simulink model "Lab2_simulink_plc"  (R2023b)
settings: Solver=FixedStepAuto  StartTime=0.0  StopTime=inf  RelTol=1e-3
InitFcn:
  | d13_init.PRE = 50;
undefined symbols (not defined in the file or in its folder): BOOL, DINT, OTL, slplc
libraries: arduinolib x9
(part 6 of 31)

== system Lab2_simulink_plc/PLC Controller Suite/__Logic/IR sampling/IR sampling/__Logic ==
  note: Continuosly sample the IR sensor
  Add Rungs : Subsystem  DisableCoverage=on  TreatAsAtomicUnit=on  mask{PLCRungNumberToAdd=2, PLCBlockType=AddRungs}
      mask prompts: PLCRungNumberToAdd "Rung Number:"
      mask Help: web([docroot '/plccoder/ref/addrungs' '.html']);
      mask DialogControl PLCAddRungs Prompt: Add Rungs
      mask DialogControl PLCRungAddButton Prompt: Add
      mask DialogControl PLCRungAddButton Callback: slplc.callbacks.addLadderRungs(gcb);
      mask Display: disp(sprintf(['Add' newline 'Rungs']));
  Add Single Rung : Subsystem  DisableCoverage=on  TreatAsAtomicUnit=on  mask{PLCRungNumberToAdd=1, PLCBlockType=AddSingleRung}
      settings: OpenFcn=slplc.callbacks.addLadderRungs(gcb);
      mask Help: web([docroot '/plccoder/ref/addsinglerung' '.html']);
      mask DialogControl PLCAddRung Prompt: Add Single Rung
      mask Display: disp(sprintf(['Add' newline '1 Rung']));
  MOV : Subsystem  Priority=3  TreatAsAtomicUnit=on  mask{PLCBlockType=MOV, PLCPOUType=stdFC}
      mask Help: web([docroot '/plccoder/ref/mov' '.html']);
      mask DialogControl PLCBlockTitle Prompt: Move (MOV)
      mask DialogControl PLCBlockDesc Prompt: Move a copy of the Source to the Destination. The Source remains unchanged.
      mask Display: disp('MOV');
  Power Rail Start : Subsystem  Priority=1  DisableCoverage=on  TreatAsAtomicUnit=on  mask{PLCBlockType=PowerRailStart}
      mask Help: web([docroot '/plccoder/ref/powerrailstart' '.html']);
      mask DialogControl PLCBlockTitle Prompt: Power Rail Start
      mask DialogControl PLCBlockDesc Prompt: This is the ladder diagram left power rail start block.
  Power Rail Terminal1 : Subsystem  DisableCoverage=on  TreatAsAtomicUnit=on  mask{PLCBlockType=PowerRailTerminal}
      mask Help: web([docroot '/plccoder/ref/powerrailterminal' '.html']);
      mask DialogControl PLCBlockTitle Prompt: Power Rail Terminal
      mask DialogControl PLCBlockDesc Prompt: This is the ladder diagram left power rail terminal block.
  Program Variables : Subsystem  Tag=ProgramVariableSS  DisableCoverage=on  TreatAsAtomicUnit=on  mask{PLCVarIR_valueName=IR_value, PLCVarIR_valueScope=External, PLCVarIR_valuePortType=Hidden, PLCVarIR_valuePortIndex=2, PLCVarIR_valueDataType=DINT, PLCVarIR_valueSize=-1, PLCVarIR_valueInitialValue=0, PLCVarIR_valueToDelete=off, PLCVarIR_value_copyName=IR_value_copy, PLCVarIR_value_copyScope=External, PLCVarIR_value_copyPortType=Hidden, PLCVarIR_value_copyPortIndex=2, PLCVarIR_value_copyDataType=DINT, PLCVarIR_value_copySize=-1, PLCVarIR_value_copyInitialValue=0, PLCVarIR_value_copyToDelete=off}
      mask init:
      | slplc.callbacks.initPOUVarSS(gcb);
      settings: OpenFcn=slplc.callbacks.openProgramVariableSS(gcb);
      mask Help: web([docroot '/plccoder/ref/programvariables' '.html']);
      mask TypeOptions PLCVarIR_valueScope: Local | External
      mask Callback PLCVarIR_valueScope: slplc.callbacks.updatePOUVarSS(gcb);
      mask TypeOptions PLCVarIR_valuePortType: Hidden | Inport | Outport
      mask Callback PLCVarIR_valuePortType: slplc.callbacks.updatePOUVarSS(gcb);
      mask TypeOptions PLCVarIR_valuePortIndex: 1 | 2
      mask TypeOptions PLCVarIR_value_copyScope: Local | External
      mask Callback PLCVarIR_value_copyScope: slplc.callbacks.updatePOUVarSS(gcb);
      mask TypeOptions PLCVarIR_value_copyPortType: Hidden | Inport | Outport
      mask Callback PLCVarIR_value_copyPortType: slplc.callbacks.updatePOUVarSS(gcb);
      mask TypeOptions PLCVarIR_value_copyPortIndex: 1 | 2
      mask DialogControl PLCBlockTitle Prompt: Program Variable Spreadsheet
      mask DialogControl PLCVarListHeaderName Prompt: Name
      mask DialogControl PLCVarListHeaderScope Prompt: Scope
      mask DialogControl PLCVarListHeaderDataType Prompt: Data Type
      mask DialogControl PLCVarListHeaderSize Prompt: Size
      mask DialogControl PLCVarListHeaderInitialValue Prompt: Initial Value
      mask DialogControl PLCVarListHeaderToDelete Prompt: Delete
  Rung Terminal1 : Subsystem  Priority=5  DisableCoverage=on  TreatAsAtomicUnit=on  mask{PLCRungTerminalIndex=1, PLCBlockType=RungTerminal}
      settings: AttributesFormatString=Rung %<PLCRungTerminalIndex>
      mask Help: web([docroot '/plccoder/ref/rungterminal' '.html']);
      mask DialogControl PLCBlockTitle Prompt: Rung Terminal
      mask DialogControl PLCBlockDescText Prompt: This is the ladder diagram rung terminal.
  Up to Parent POU : Subsystem  DisableCoverage=on  TreatAsAtomicUnit=on  mask{PLCBlockType=OpenParentPOU}
      settings: OpenFcn=slplc.callbacks.openParentPOUBlock(gcb);
      mask Help: web([docroot '/plccoder/ref/uptoparentpou' '.html']);
      mask DialogControl PLCBlockTitle Prompt: Add Single Rung
  Variable Read : Subsystem  Priority=2  TreatAsAtomicUnit=on  mask{PLCOperandTag=IR_value, PLCBlockType=VariableRead, PLCOperandAccess=Read, PLCPOUType=VariableRead, PLCOperandExpression=xxx_PLC_VAR_IR_value}
      mask prompts: PLCOperandTag "Data Tag:"
      mask init:
      | block = gcb;
      | maskObj = Simulink.Mask.get(block);
      | maskObj.Display = sprintf('disp(''%s'');', PLCOperandTag);
      | slplc.callbacks.initInstruction(block);
      mask Help: web([docroot '/plccoder/ref/variableread' '.html']);
      mask DialogControl PLCBlockTitle Prompt: Data Tag Read
      mask Display: disp('IR_value');
  Variable Write : Subsystem  Priority=4  TreatAsAtomicUnit=on  mask{PLCOperandTag=IR_value_copy, PLCBlockType=VariableWrite, PLCOperandAccess=Write, PLCPOUType=VariableWrite, PLCOperandExpression=xxx_PLC_VAR_IR_value_copy}
      mask prompts: PLCOperandTag "Data Tag:"
      mask init: same as Lab2_simulink_plc/PLC Controller Suite/__Logic/Fukt logic/Program/__Logic/Variable Write
      mask Help: web([docroot '/plccoder/ref/variablewrite' '.html']);
      mask DialogControl PLCBlockTitle Prompt: Data Tag Write
      mask Display: disp('IR_value_copy');
  wires:
  Power Rail Start/1(PowerOut) -> Power Rail Terminal1/1(PowerIn), MOV/1(RungIn)
  MOV/1(RungOut) -> Rung Terminal1/1(RungIn)
  Variable Read/1(VarOut) -> MOV/2(src)
  MOV/2(dest) -> Variable Write/1(VarIn)

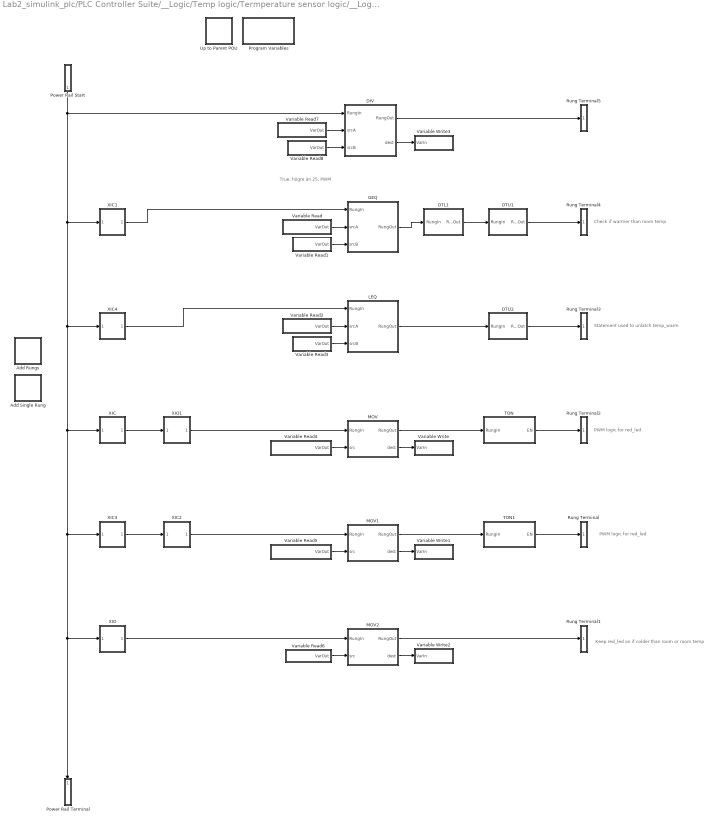
== system Lab2_simulink_plc/PLC Controller Suite/__Logic/Temp logic/Termperature sensor logic/__Logic ==
  note: Keep red_led on if colder than room or room temp
  note: PWM logic for red_led
  note: Check if warmer than room temp
  note: Statement used to unlatch temp_warm
  note: True, högre än 25, PWM
  note: PWM logic for red_led
  Add Rungs : Subsystem  DisableCoverage=on  TreatAsAtomicUnit=on  mask{PLCRungNumberToAdd=2, PLCBlockType=AddRungs}
      mask prompts: PLCRungNumberToAdd "Rung Number:"
      mask Help: web([docroot '/plccoder/ref/addrungs' '.html']);
      mask DialogControl PLCAddRungs Prompt: Add Rungs
      mask DialogControl PLCRungAddButton Prompt: Add
      mask DialogControl PLCRungAddButton Callback: slplc.callbacks.addLadderRungs(gcb);
      mask Display: disp(sprintf(['Add' newline 'Rungs']));
  Add Single Rung : Subsystem  DisableCoverage=on  TreatAsAtomicUnit=on  mask{PLCRungNumberToAdd=1, PLCBlockType=AddSingleRung}
      settings: OpenFcn=slplc.callbacks.addLadderRungs(gcb);
      mask Help: web([docroot '/plccoder/ref/addsinglerung' '.html']);
      mask DialogControl PLCAddRung Prompt: Add Single Rung
      mask Display: disp(sprintf(['Add' newline '1 Rung']));
  DIV : Subsystem  Priority=4  TreatAsAtomicUnit=on  mask{PLCBlockType=DIV, PLCPOUType=stdFC}
      mask Help: web([docroot '/plccoder/ref/div' '.html']);
      mask DialogControl PLCBlockTitle Prompt: Divide (DIV)
      mask DialogControl PLCBlockDesc Prompt: Divide Source A by Source B.
      mask Display: disp('DIV');
  GEQ : Subsystem  Priority=10  TreatAsAtomicUnit=on  mask{PLCBlockType=GEQ, PLCPOUType=stdFC}
      mask Help: web([docroot '/plccoder/ref/geq' '.html']);
      mask DialogControl PLCBlockTitle Prompt: Greater Than or Equal To (GEQ)
      mask DialogControl PLCBlockDesc Prompt: Test whether Source A is greater than or equal to Source B.
      mask Display: disp('GEQ');
  LEQ : Subsystem  Priority=17  TreatAsAtomicUnit=on  mask{PLCBlockType=LEQ, PLCPOUType=stdFC}
      mask Help: web([docroot '/plccoder/ref/leq' '.html']);
      mask DialogControl PLCBlockTitle Prompt: Less Than or Equal To (LEQ)
      mask DialogControl PLCBlockDesc Prompt: Test whether Source A is less than or equal to Source B.
      mask Display: disp('LEQ');
  MOV : Subsystem  Priority=23  TreatAsAtomicUnit=on  mask{PLCBlockType=MOV, PLCPOUType=stdFC}
      mask Help: web([docroot '/plccoder/ref/mov' '.html']);
      mask DialogControl PLCBlockTitle Prompt: Move (MOV)
      mask DialogControl PLCBlockDesc Prompt: Move a copy of the Source to the Destination. The Source remains unchanged.
      mask Display: disp('MOV');
  MOV1 : Subsystem  Priority=30  TreatAsAtomicUnit=on  mask{PLCBlockType=MOV, PLCPOUType=stdFC}
      mask Help: web([docroot '/plccoder/ref/mov' '.html']);
      mask DialogControl PLCBlockTitle Prompt: Move (MOV)
      mask DialogControl PLCBlockDesc Prompt: Move a copy of the Source to the Destination. The Source remains unchanged.
      mask Display: disp('MOV');
  MOV2 : Subsystem  Priority=36  TreatAsAtomicUnit=on  mask{PLCBlockType=MOV, PLCPOUType=stdFC}
      mask Help: web([docroot '/plccoder/ref/mov' '.html']);
      mask DialogControl PLCBlockTitle Prompt: Move (MOV)
      mask DialogControl PLCBlockDesc Prompt: Move a copy of the Source to the Destination. The Source remains unchanged.
      mask Display: disp('MOV');
  OTL1 : Subsystem  Priority=11  TreatAsAtomicUnit=on  mask{PLCOperandTag=temp_warm, PLCBlockType=OTL, PLCPOUType=Coil, PLCOperandExpression=xxx_PLC_VAR_temp_warm}
      mask prompts: PLCOperandTag "Operand Tag:"
      mask init:
      | slplc.callbacks.initInstruction(gcb);
      settings: AttributesFormatString=%<PLCOperandTag>
      mask Help: web([docroot '/plccoder/ref/otl' '.html']);
      mask DialogControl PLCBlockTitle Prompt: Output Latch (OTL)
      mask Display: disp('L');
  OTU1 : Subsystem  Priority=12  TreatAsAtomicUnit=on  mask{PLCOperandTag=TempPolling, PLCBlockType=OTU, PLCPOUType=Coil, PLCOperandExpression=xxx_PLC_VAR_TempPolling}
      mask prompts: PLCOperandTag "Operand Tag:"
      mask init:
      | slplc.callbacks.initInstruction(gcb);
      settings: AttributesFormatString=%<PLCOperandTag>
      mask Help: web([docroot '/plccoder/ref/otu' '.html']);
      mask DialogControl PLCBlockTitle Prompt: Output Unlatch (OTU)
      mask Display: disp('U');
  OTU2 : Subsystem  Priority=18  TreatAsAtomicUnit=on  mask{PLCOperandTag=temp_warm, PLCBlockType=OTU, PLCPOUType=Coil, PLCOperandExpression=xxx_PLC_VAR_temp_warm}
      mask prompts: PLCOperandTag "Operand Tag:"
      mask init:
      | slplc.callbacks.initInstruction(gcb);
      settings: AttributesFormatString=%<PLCOperandTag>
      mask Help: web([docroot '/plccoder/ref/otu' '.html']);
      mask DialogControl PLCBlockTitle Prompt: Output Unlatch (OTU)
      mask Display: disp('U');
  Power Rail Start : Subsystem  Priority=1  DisableCoverage=on  TreatAsAtomicUnit=on  mask{PLCBlockType=PowerRailStart}
      mask Help: web([docroot '/plccoder/ref/powerrailstart' '.html']);
      mask DialogControl PLCBlockTitle Prompt: Power Rail Start
      mask DialogControl PLCBlockDesc Prompt: This is the ladder diagram left power rail start block.
  Power Rail Terminal : Subsystem  Priority=14  DisableCoverage=on  TreatAsAtomicUnit=on  mask{PLCBlockType=PowerRailTerminal}
      mask Help: web([docroot '/plccoder/ref/powerrailterminal' '.html']);
      mask DialogControl PLCBlockTitle Prompt: Power Rail Terminal
      mask DialogControl PLCBlockDesc Prompt: This is the ladder diagram left power rail terminal block.
  Program Variables : Subsystem  Tag=ProgramVariableSS  DisableCoverage=on  TreatAsAtomicUnit=on  mask{PLCVarRoom_tempName=Room_temp, PLCVarRoom_tempScope=Local, PLCVarRoom_tempPortType=Hidden, PLCVarRoom_tempPortIndex=10, PLCVarRoom_tempDataType=uint16, PLCVarRoom_tempSize=1, PLCVarRoom_tempInitialValue=150, PLCVarRoom_tempToDelete=off, PLCVarT1Name=T1, PLCVarT1Scope=Local, PLCVarT1PortType=Hidden, PLCVarT1PortIndex=10, PLCVarT1DataType=Bus: TIMER, PLCVarT1Size=1, PLCVarT1InitialValue=red_period, PLCVarT1ToDelete=off, PLCVarT2Name=T2, PLCVarT2Scope=Local, PLCVarT2PortType=Hidden, PLCVarT2PortIndex=10, PLCVarT2DataType=Bus: TIMER, PLCVarT2Size=1, PLCVarT2InitialValue=red_period, PLCVarT2ToDelete=off, PLCVarconstantName=constant, PLCVarconstantScope=Local, PLCVarconstantPortType=Hidden, PLCVarconstantPortIndex=10, PLCVarconstantDataType=uint16, PLCVarconstantSize=1, PLCVarconstantInitialValue=3, PLCVarconstantToDelete=off, PLCVarduty_cycleName=duty_cycle, PLCVarduty_cycleScope=Local, PLCVarduty_cyclePortType=Hidden, PLCVarduty_cyclePortIndex=10, PLCVarduty_cycleDataType=uint8, PLCVarduty_cycleSize=1, PLCVarduty_cycleInitialValue=0, PLCVarduty_cycleToDelete=off, PLCVarred_led_offName=red_led_off, PLCVarred_led_offScope=Local, PLCVarred_led_offPortType=Hidden, PLCVarred_led_offPortIndex=10, PLCVarred_led_offDataType=uint8, PLCVarred_led_offSize=1, PLCVarred_led_offInitialValue=0, PLCVarred_led_offToDelete=off, PLCVarRedLedName=RedLed, PLCVarRedLedScope=External, PLCVarRedLedPortType=Hidden, PLCVarRedLedPortIndex=10, PLCVarRedLedDataType=DINT, PLCVarRedLedSize=-1, PLCVarRedLedInitialValue=0, PLCVarRedLedToDelete=off, PLCVarTempPollingName=TempPolling, PLCVarTempPollingScope=External, PLCVarTempPollingPortType=Hidden, PLCVarTempPollingPortIndex=10, PLCVarTempPollingDataType=DINT, PLCVarTempPollingSize=-1, PLCVarTempPollingInitialValue=0, PLCVarTempPollingToDelete=off, PLCVartemp_sensor_copyName=temp_sensor_copy, PLCVartemp_sensor_copyScope=External, PLCVartemp_sensor_copyPortType=Hidden, PLCVartemp_sensor_copyPortIndex=10, PLCVartemp_sensor_copyDataType=DINT, PLCVartemp_sensor_copySize=-1, PLCVartemp_sensor_copyInitialValue=0, PLCVartemp_sensor_copyToDelete=off, PLCVartemp_warmName=temp_warm, PLCVartemp_warmScope=External, PLCVartemp_warmPortType=Hidden, PLCVartemp_warmPortIndex=10, PLCVartemp_warmDataType=DINT, PLCVartemp_warmSize=-1, PLCVartemp_warmInitialValue=0, PLCVartemp_warmToDelete=off}
      mask init:
      | slplc.callbacks.initPOUVarSS(gcb);
      settings: OpenFcn=slplc.callbacks.openProgramVariableSS(gcb);
      mask Help: web([docroot '/plccoder/ref/programvariables' '.html']);
      mask TypeOptions PLCVarRoom_tempScope: Local | External
      mask Callback PLCVarRoom_tempScope: slplc.callbacks.updatePOUVarSS(gcb);
      mask TypeOptions PLCVarRoom_tempPortType: Hidden | Inport | Outport
      mask Callback PLCVarRoom_tempPortType: slplc.callbacks.updatePOUVarSS(gcb);
      mask TypeOptions PLCVarRoom_tempPortIndex: 1 | 2 | 3 | 4 | 5 | 6 | 7 | 8 | 9 | 10
      mask TypeOptions PLCVarT1Scope: Local | External
      mask Callback PLCVarT1Scope: slplc.callbacks.updatePOUVarSS(gcb);
      mask TypeOptions PLCVarT1PortType: Hidden | Inport | Outport
      mask Callback PLCVarT1PortType: slplc.callbacks.updatePOUVarSS(gcb);
      mask TypeOptions PLCVarT1PortIndex: 1 | 2 | 3 | 4 | 5 | 6 | 7 | 8 | 9 | 10
      mask TypeOptions PLCVarT2Scope: Local | External
      mask Callback PLCVarT2Scope: slplc.callbacks.updatePOUVarSS(gcb);
      mask TypeOptions PLCVarT2PortType: Hidden | Inport | Outport
      mask Callback PLCVarT2PortType: slplc.callbacks.updatePOUVarSS(gcb);
      mask TypeOptions PLCVarT2PortIndex: 1 | 2 | 3 | 4 | 5 | 6 | 7 | 8 | 9 | 10
      mask TypeOptions PLCVarconstantScope: Local | External
      mask Callback PLCVarconstantScope: slplc.callbacks.updatePOUVarSS(gcb);
      mask TypeOptions PLCVarconstantPortType: Hidden | Inport | Outport
      mask Callback PLCVarconstantPortType: slplc.callbacks.updatePOUVarSS(gcb);
      mask TypeOptions PLCVarconstantPortIndex: 1 | 2 | 3 | 4 | 5 | 6 | 7 | 8 | 9 | 10
      mask TypeOptions PLCVarduty_cycleScope: Local | External
      mask Callback PLCVarduty_cycleScope: slplc.callbacks.updatePOUVarSS(gcb);
      mask TypeOptions PLCVarduty_cyclePortType: Hidden | Inport | Outport
      mask Callback PLCVarduty_cyclePortType: slplc.callbacks.updatePOUVarSS(gcb);
      mask TypeOptions PLCVarduty_cyclePortIndex: 1 | 2 | 3 | 4 | 5 | 6 | 7 | 8 | 9 | 10
      mask TypeOptions PLCVarred_led_offScope: Local | External
      mask Callback PLCVarred_led_offScope: slplc.callbacks.updatePOUVarSS(gcb);
      mask TypeOptions PLCVarred_led_offPortType: Hidden | Inport | Outport
      mask Callback PLCVarred_led_offPortType: slplc.callbacks.updatePOUVarSS(gcb);
      mask TypeOptions PLCVarred_led_offPortIndex: 1 | 2 | 3 | 4 | 5 | 6 | 7 | 8 | 9 | 10
      mask TypeOptions PLCVarRedLedScope: Local | External
      mask Callback PLCVarRedLedScope: slplc.callbacks.updatePOUVarSS(gcb);
      mask TypeOptions PLCVarRedLedPortType: Hidden | Inport | Outport
      mask Callback PLCVarRedLedPortType: slplc.callbacks.updatePOUVarSS(gcb);
      mask TypeOptions PLCVarRedLedPortIndex: 1 | 2 | 3 | 4 | 5 | 6 | 7 | 8 | 9 | 10
      mask TypeOptions PLCVarTempPollingScope: Local | External
      mask Callback PLCVarTempPollingScope: slplc.callbacks.updatePOUVarSS(gcb);
      mask TypeOptions PLCVarTempPollingPortType: Hidden | Inport | Outport
      mask Callback PLCVarTempPollingPortType: slplc.callbacks.updatePOUVarSS(gcb);
      mask TypeOptions PLCVarTempPollingPortIndex: 1 | 2 | 3 | 4 | 5 | 6 | 7 | 8 | 9 | 10
      mask TypeOptions PLCVartemp_sensor_copyScope: Local | External
      mask Callback PLCVartemp_sensor_copyScope: slplc.callbacks.updatePOUVarSS(gcb);
      mask TypeOptions PLCVartemp_sensor_copyPortType: Hidden | Inport | Outport
      mask Callback PLCVartemp_sensor_copyPortType: slplc.callbacks.updatePOUVarSS(gcb);
      mask TypeOptions PLCVartemp_sensor_copyPortIndex: 1 | 2 | 3 | 4 | 5 | 6 | 7 | 8 | 9 | 10
      mask TypeOptions PLCVartemp_warmScope: Local | External
      mask Callback PLCVartemp_warmScope: slplc.callbacks.updatePOUVarSS(gcb);
      mask TypeOptions PLCVartemp_warmPortType: Hidden | Inport | Outport
      mask Callback PLCVartemp_warmPortType: slplc.callbacks.updatePOUVarSS(gcb);
      mask TypeOptions PLCVartemp_warmPortIndex: 1 | 2 | 3 | 4 | 5 | 6 | 7 | 8 | 9 | 10
      mask DialogControl PLCBlockTitle Prompt: Program Variable Spreadsheet
      mask DialogControl PLCVarListHeaderName Prompt: Name
      mask DialogControl PLCVarListHeaderScope Prompt: Scope
      mask DialogControl PLCVarListHeaderDataType Prompt: Data Type
      mask DialogControl PLCVarListHeaderSize Prompt: Size
      mask DialogControl PLCVarListHeaderInitialValue Prompt: Initial Value
      mask DialogControl PLCVarListHeaderToDelete Prompt: Delete
  Rung Terminal : Subsystem  Priority=33  DisableCoverage=on  TreatAsAtomicUnit=on  mask{PLCRungTerminalIndex=5, PLCBlockType=RungTerminal}
      settings: AttributesFormatString=Rung %<PLCRungTerminalIndex>
      mask Help: web([docroot '/plccoder/ref/rungterminal' '.html']);
      mask DialogControl PLCBlockTitle Prompt: Rung Terminal
      mask DialogControl PLCBlockDescText Prompt: This is the ladder diagram rung terminal.
  Rung Terminal1 : Subsystem  Priority=38  DisableCoverage=on  TreatAsAtomicUnit=on  mask{PLCRungTerminalIndex=6, PLCBlockType=RungTerminal}
      settings: AttributesFormatString=Rung %<PLCRungTerminalIndex>
      mask Help: web([docroot '/plccoder/ref/rungterminal' '.html']);
      mask DialogControl PLCBlockTitle Prompt: Rung Terminal
      mask DialogControl PLCBlockDescText Prompt: This is the ladder diagram rung terminal.
  Rung Terminal2 : Subsystem  Priority=26  DisableCoverage=on  TreatAsAtomicUnit=on  mask{PLCRungTerminalIndex=4, PLCBlockType=RungTerminal}
      settings: AttributesFormatString=Rung %<PLCRungTerminalIndex>
      mask Help: web([docroot '/plccoder/ref/rungterminal' '.html']);
      mask DialogControl PLCBlockTitle Prompt: Rung Terminal
      mask DialogControl PLCBlockDescText Prompt: This is the ladder diagram rung terminal.
  Rung Terminal3 : Subsystem  Priority=19  DisableCoverage=on  TreatAsAtomicUnit=on  mask{PLCRungTerminalIndex=3, PLCBlockType=RungTerminal}
      settings: AttributesFormatString=Rung %<PLCRungTerminalIndex>
      mask Help: web([docroot '/plccoder/ref/rungterminal' '.html']);
      mask DialogControl PLCBlockTitle Prompt: Rung Terminal
      mask DialogControl PLCBlockDescText Prompt: This is the ladder diagram rung terminal.
  Rung Terminal4 : Subsystem  Priority=13  DisableCoverage=on  TreatAsAtomicUnit=on  mask{PLCRungTerminalIndex=2, PLCBlockType=RungTerminal}
      settings: AttributesFormatString=Rung %<PLCRungTerminalIndex>
      mask Help: web([docroot '/plccoder/ref/rungterminal' '.html']);
      mask DialogControl PLCBlockTitle Prompt: Rung Terminal
      mask DialogControl PLCBlockDescText Prompt: This is the ladder diagram rung terminal.
  Rung Terminal5 : Subsystem  Priority=6  DisableCoverage=on  TreatAsAtomicUnit=on  mask{PLCRungTerminalIndex=1, PLCBlockType=RungTerminal}
      settings: AttributesFormatString=Rung %<PLCRungTerminalIndex>
      mask Help: web([docroot '/plccoder/ref/rungterminal' '.html']);
      mask DialogControl PLCBlockTitle Prompt: Rung Terminal
      mask DialogControl PLCBlockDescText Prompt: This is the ladder diagram rung terminal.
  TON : Subsystem  Priority=25  TreatAsAtomicUnit=on  mask{PLCOperandTag=T1, PLCTimerSampleTime=0.2, PLCBlockType=TON, PLCPOUType=stdFB, PLCOperandAccess=ReadWrite, PLCOperandExpression=xxx_PLC_VAR_T1}
      mask prompts: PLCOperandTag "Timer Tag:"; PLCTimerSampleTime "Timer Sample Time:"
      mask init:
      | block = gcb;
      | maskObj = Simulink.Mask.get(block);
      | maskObj.Display = sprintf('disp([''%s'' newline newline ''%s'']);', PLCBlockType, PLCOperandTag);
      | slplc.callbacks.initInstruction(block);
      settings: AttributesFormatString=%<PLCBlockType>: %<PLCOperandTag>
      mask Help: web([docroot '/plccoder/ref/ton' '.html']);
      mask DialogControl PLCBlockTitle Prompt: Timer On Delay (TON)
      mask Display: disp(['TON' newline newline 'T1']);
  TON1 : Subsystem  Priority=32  TreatAsAtomicUnit=on  mask{PLCOperandTag=T2, PLCTimerSampleTime=0.2, PLCBlockType=TON, PLCPOUType=stdFB, PLCOperandAccess=ReadWrite, PLCOperandExpression=xxx_PLC_VAR_T2}
      mask prompts: PLCOperandTag "Timer Tag:"; PLCTimerSampleTime "Timer Sample Time:"
      mask init:
      | block = gcb;
      | maskObj = Simulink.Mask.get(block);
      | maskObj.Display = sprintf('disp([''%s'' newline newline ''%s'']);', PLCBlockType, PLCOperandTag);
      | slplc.callbacks.initInstruction(block);
      settings: AttributesFormatString=%<PLCBlockType>: %<PLCOperandTag>
      mask Help: web([docroot '/plccoder/ref/ton' '.html']);
      mask DialogControl PLCBlockTitle Prompt: Timer On Delay (TON)
      mask Display: disp(['TON' newline newline 'T2']);
  Up to Parent POU : Subsystem  DisableCoverage=on  TreatAsAtomicUnit=on  mask{PLCBlockType=OpenParentPOU}
      settings: OpenFcn=slplc.callbacks.openParentPOUBlock(gcb);
      mask Help: web([docroot '/plccoder/ref/uptoparentpou' '.html']);
      mask DialogControl PLCBlockTitle Prompt: Add Single Rung
  Variable Read : Subsystem  Priority=8  TreatAsAtomicUnit=on  mask{PLCOperandTag=temp_sensor_copy, PLCBlockType=VariableRead, PLCOperandAccess=Read, PLCPOUType=VariableRead, PLCOperandExpression=xxx_PLC_VAR_temp_sensor_copy}
      mask prompts: PLCOperandTag "Data Tag:"
      mask init:
      | block = gcb;
      | maskObj = Simulink.Mask.get(block);
      | maskObj.Display = sprintf('disp(''%s'');', PLCOperandTag);
      | slplc.callbacks.initInstruction(block);
      mask Help: web([docroot '/plccoder/ref/variableread' '.html']);
      mask DialogControl PLCBlockTitle Prompt: Data Tag Read
      mask Display: disp('temp_sensor_copy');
  Variable Read1 : Subsystem  Priority=9  TreatAsAtomicUnit=on  mask{PLCOperandTag=Room_temp, PLCBlockType=VariableRead, PLCOperandAccess=Read, PLCPOUType=VariableRead, PLCOperandExpression=xxx_PLC_VAR_Room_temp}
      mask prompts: PLCOperandTag "Data Tag:"
      mask init:
      | block = gcb;
      | maskObj = Simulink.Mask.get(block);
      | maskObj.Display = sprintf('disp(''%s'');', PLCOperandTag);
      | slplc.callbacks.initInstruction(block);
      mask Help: web([docroot '/plccoder/ref/variableread' '.html']);
      mask DialogControl PLCBlockTitle Prompt: Data Tag Read
      mask Display: disp('Room_temp');
  Variable Read2 : Subsystem  Priority=15  TreatAsAtomicUnit=on  mask{PLCOperandTag=temp_sensor_copy, PLCBlockType=VariableRead, PLCOperandAccess=Read, PLCPOUType=VariableRead, PLCOperandExpression=xxx_PLC_VAR_temp_sensor_copy}
      mask prompts: PLCOperandTag "Data Tag:"
      mask init:
      | block = gcb;
      | maskObj = Simulink.Mask.get(block);
      | maskObj.Display = sprintf('disp(''%s'');', PLCOperandTag);
      | slplc.callbacks.initInstruction(block);
      mask Help: web([docroot '/plccoder/ref/variableread' '.html']);
      mask DialogControl PLCBlockTitle Prompt: Data Tag Read
      mask Display: disp('temp_sensor_copy');
  Variable Read3 : Subsystem  Priority=16  TreatAsAtomicUnit=on  mask{PLCOperandTag=Room_temp, PLCBlockType=VariableRead, PLCOperandAccess=Read, PLCPOUType=VariableRead, PLCOperandExpression=xxx_PLC_VAR_Room_temp}
      mask prompts: PLCOperandTag "Data Tag:"
      mask init:
      | block = gcb;
      | maskObj = Simulink.Mask.get(block);
      | maskObj.Display = sprintf('disp(''%s'');', PLCOperandTag);
      | slplc.callbacks.initInstruction(block);
      mask Help: web([docroot '/plccoder/ref/variableread' '.html']);
      mask DialogControl PLCBlockTitle Prompt: Data Tag Read
      mask Display: disp('Room_temp');
  Variable Read4 : Subsystem  Priority=22  TreatAsAtomicUnit=on  mask{PLCOperandTag=duty_cycle, PLCBlockType=VariableRead, PLCOperandAccess=Read, PLCPOUType=VariableRead, PLCOperandExpression=xxx_PLC_VAR_duty_cycle}
      mask prompts: PLCOperandTag "Data Tag:"
      mask init:
      | block = gcb;
      | maskObj = Simulink.Mask.get(block);
      | maskObj.Display = sprintf('disp(''%s'');', PLCOperandTag);
      | slplc.callbacks.initInstruction(block);
      mask Help: web([docroot '/plccoder/ref/variableread' '.html']);
      mask DialogControl PLCBlockTitle Prompt: Data Tag Read
      mask Display: disp('duty_cycle');
  Variable Read5 : Subsystem  Priority=29  TreatAsAtomicUnit=on  mask{PLCOperandTag=red_led_off, PLCBlockType=VariableRead, PLCOperandAccess=Read, PLCPOUType=VariableRead, PLCOperandExpression=xxx_PLC_VAR_red_led_off}
      mask prompts: PLCOperandTag "Data Tag:"
      mask init:
      | block = gcb;
      | maskObj = Simulink.Mask.get(block);
      | maskObj.Display = sprintf('disp(''%s'');', PLCOperandTag);
      | slplc.callbacks.initInstruction(block);
      mask Help: web([docroot '/plccoder/ref/variableread' '.html']);
      mask DialogControl PLCBlockTitle Prompt: Data Tag Read
      mask Display: disp('red_led_off');
  Variable Read6 : Subsystem  Priority=35  TreatAsAtomicUnit=on  mask{PLCOperandTag=duty_cycle, PLCBlockType=VariableRead, PLCOperandAccess=Read, PLCPOUType=VariableRead, PLCOperandExpression=xxx_PLC_VAR_duty_cycle}
      mask prompts: PLCOperandTag "Data Tag:"
      mask init:
      | block = gcb;
      | maskObj = Simulink.Mask.get(block);
      | maskObj.Display = sprintf('disp(''%s'');', PLCOperandTag);
      | slplc.callbacks.initInstruction(block);
      mask Help: web([docroot '/plccoder/ref/variableread' '.html']);
      mask DialogControl PLCBlockTitle Prompt: Data Tag Read
      mask Display: disp('duty_cycle');
  Variable Read7 : Subsystem  Priority=2  TreatAsAtomicUnit=on  mask{PLCOperandTag=temp_sensor_copy, PLCBlockType=VariableRead, PLCOperandAccess=Read, PLCPOUType=VariableRead, PLCOperandExpression=xxx_PLC_VAR_temp_sensor_copy}
      mask prompts: PLCOperandTag "Data Tag:"
      mask init:
      | block = gcb;
      | maskObj = Simulink.Mask.get(block);
      | maskObj.Display = sprintf('disp(''%s'');', PLCOperandTag);
      | slplc.callbacks.initInstruction(block);
      mask Help: web([docroot '/plccoder/ref/variableread' '.html']);
      mask DialogControl PLCBlockTitle Prompt: Data Tag Read
      mask Display: disp('temp_sensor_copy');
  Variable Read8 : Subsystem  Priority=3  TreatAsAtomicUnit=on  mask{PLCOperandTag=constant, PLCBlockType=VariableRead, PLCOperandAccess=Read, PLCPOUType=VariableRead, PLCOperandExpression=xxx_PLC_VAR_constant}
      mask prompts: PLCOperandTag "Data Tag:"
      mask init:
      | block = gcb;
      | maskObj = Simulink.Mask.get(block);
      | maskObj.Display = sprintf('disp(''%s'');', PLCOperandTag);
      | slplc.callbacks.initInstruction(block);
      mask Help: web([docroot '/plccoder/ref/variableread' '.html']);
      mask DialogControl PLCBlockTitle Prompt: Data Tag Read
      mask Display: disp('constant');
  Variable Write : Subsystem  Priority=24  TreatAsAtomicUnit=on  mask{PLCOperandTag=RedLed, PLCBlockType=VariableWrite, PLCOperandAccess=Write, PLCPOUType=VariableWrite, PLCOperandExpression=xxx_PLC_VAR_RedLed}
      mask prompts: PLCOperandTag "Data Tag:"
      mask init: same as Lab2_simulink_plc/PLC Controller Suite/__Logic/Fukt logic/Program/__Logic/Variable Write
      mask Help: web([docroot '/plccoder/ref/variablewrite' '.html']);
      mask DialogControl PLCBlockTitle Prompt: Data Tag Write
      mask Display: disp('RedLed');
  Variable Write1 : Subsystem  Priority=31  TreatAsAtomicUnit=on  mask{PLCOperandTag=RedLed, PLCBlockType=VariableWrite, PLCOperandAccess=Write, PLCPOUType=VariableWrite, PLCOperandExpression=xxx_PLC_VAR_RedLed}
      mask prompts: PLCOperandTag "Data Tag:"
      mask init: same as Lab2_simulink_plc/PLC Controller Suite/__Logic/Fukt logic/Program/__Logic/Variable Write
      mask Help: web([docroot '/plccoder/ref/variablewrite' '.html']);
      mask DialogControl PLCBlockTitle Prompt: Data Tag Write
      mask Display: disp('RedLed');
  Variable Write2 : Subsystem  Priority=37  TreatAsAtomicUnit=on  mask{PLCOperandTag=RedLed, PLCBlockType=VariableWrite, PLCOperandAccess=Write, PLCPOUType=VariableWrite, PLCOperandExpression=xxx_PLC_VAR_RedLed}
      mask prompts: PLCOperandTag "Data Tag:"
      mask init: same as Lab2_simulink_plc/PLC Controller Suite/__Logic/Fukt logic/Program/__Logic/Variable Write
      mask Help: web([docroot '/plccoder/ref/variablewrite' '.html']);
      mask DialogControl PLCBlockTitle Prompt: Data Tag Write
      mask Display: disp('RedLed');
  Variable Write3 : Subsystem  Priority=5  TreatAsAtomicUnit=on  mask{PLCOperandTag=duty_cycle, PLCBlockType=VariableWrite, PLCOperandAccess=Write, PLCPOUType=VariableWrite, PLCOperandExpression=xxx_PLC_VAR_duty_cycle}
      mask prompts: PLCOperandTag "Data Tag:"
      mask init: same as Lab2_simulink_plc/PLC Controller Suite/__Logic/Fukt logic/Program/__Logic/Variable Write
      mask Help: web([docroot '/plccoder/ref/variablewrite' '.html']);
      mask DialogControl PLCBlockTitle Prompt: Data Tag Write
      mask Display: disp('duty_cycle');
  XIC : Subsystem  Priority=20  TreatAsAtomicUnit=on  mask{PLCOperandTag=temp_warm, PLCBlockType=XIC, PLCPOUType=Contact, PLCOperandExpression=xxx_PLC_VAR_temp_warm}
      mask prompts: PLCOperandTag "Operand Tag:"
      mask init:
      | slplc.callbacks.initInstruction(gcb);
      settings: AttributesFormatString=%<PLCOperandTag>
      mask Help: web([docroot '/plccoder/ref/xic' '.html']);
      mask DialogControl PLCBlockTitle Prompt: Examine If Closed (XIC)
  XIC1 : Subsystem  Priority=7  TreatAsAtomicUnit=on  mask{PLCOperandTag=TempPolling, PLCBlockType=XIC, PLCPOUType=Contact, PLCOperandExpression=xxx_PLC_VAR_TempPolling}
      mask prompts: PLCOperandTag "Operand Tag:"
      mask init:
      | slplc.callbacks.initInstruction(gcb);
      settings: AttributesFormatString=%<PLCOperandTag>
      mask Help: web([docroot '/plccoder/ref/xic' '.html']);
      mask DialogControl PLCBlockTitle Prompt: Examine If Closed (XIC)
  XIC2 : Subsystem  Priority=28  TreatAsAtomicUnit=on  mask{PLCOperandTag=T1.DN, PLCBlockType=XIC, PLCPOUType=Contact, PLCOperandExpression=xxx_PLC_VAR_T1.DN}
      mask prompts: PLCOperandTag "Operand Tag:"
      mask init:
      | slplc.callbacks.initInstruction(gcb);
      settings: AttributesFormatString=%<PLCOperandTag>
      mask Help: web([docroot '/plccoder/ref/xic' '.html']);
      mask DialogControl PLCBlockTitle Prompt: Examine If Closed (XIC)
  XIC3 : Subsystem  Priority=27  TreatAsAtomicUnit=on  mask{PLCOperandTag=temp_warm, PLCBlockType=XIC, PLCPOUType=Contact, PLCOperandExpression=xxx_PLC_VAR_temp_warm}
      mask prompts: PLCOperandTag "Operand Tag:"
      mask init:
      | slplc.callbacks.initInstruction(gcb);
      settings: AttributesFormatString=%<PLCOperandTag>
      mask Help: web([docroot '/plccoder/ref/xic' '.html']);
      mask DialogControl PLCBlockTitle Prompt: Examine If Closed (XIC)
  XIC4 : Subsystem  Priority=14  TreatAsAtomicUnit=on  mask{PLCOperandTag=temp_warm, PLCBlockType=XIC, PLCPOUType=Contact, PLCOperandExpression=xxx_PLC_VAR_temp_warm}
      mask prompts: PLCOperandTag "Operand Tag:"
      mask init:
      | slplc.callbacks.initInstruction(gcb);
      settings: AttributesFormatString=%<PLCOperandTag>
      mask Help: web([docroot '/plccoder/ref/xic' '.html']);
      mask DialogControl PLCBlockTitle Prompt: Examine If Closed (XIC)
  XIO : Subsystem  Priority=34  TreatAsAtomicUnit=on  mask{PLCOperandExpression=xxx_PLC_VAR_temp_warm, PLCOperandTag=temp_warm, PLCBlockType=XIO, PLCPOUType=Contact}
      mask prompts: PLCOperandTag "Operand Tag:"
      mask init:
      | slplc.callbacks.initInstruction(gcb);
      settings: AttributesFormatString=%<PLCOperandTag>
      mask Help: web([docroot '/plccoder/ref/xio' '.html']);
      mask DialogControl PLCBlockTitle Prompt: Examine If Open (XIO)
  XIO1 : Subsystem  Priority=21  TreatAsAtomicUnit=on  mask{PLCOperandExpression=xxx_PLC_VAR_T2.DN, PLCOperandTag=T2.DN, PLCBlockType=XIO, PLCPOUType=Contact}
      mask prompts: PLCOperandTag "Operand Tag:"
      mask init:
      | slplc.callbacks.initInstruction(gcb);
      settings: AttributesFormatString=%<PLCOperandTag>
      mask Help: web([docroot '/plccoder/ref/xio' '.html']);
      mask DialogControl PLCBlockTitle Prompt: Examine If Open (XIO)
  wires:
  Variable Read/1(VarOut) -> GEQ/2(srcA)
  XIO/1(RungOut) -> MOV2/1(RungIn)
  Variable Read1/1(VarOut) -> GEQ/3(srcB)
  XIC1/1(RungOut) -> GEQ/1(RungIn)
  OTU1/1(RungOut) -> Rung Terminal4/1(RungIn)
  MOV1/1(RungOut) -> TON1/1(RungIn)
  XIC2/1(RungOut) -> MOV1/1(RungIn)
  XIC/1(RungOut) -> XIO1/1(RungIn)
  XIC3/1(RungOut) -> XIC2/1(RungIn)
  MOV2/1(RungOut) -> Rung Terminal1/1(RungIn)
  TON1/1(EN) -> Rung Terminal/1(RungIn)
  GEQ/1(RungOut) -> OTL1/1(RungIn)
  XIC4/1(RungOut) -> LEQ/1(RungIn)
  Variable Read2/1(VarOut) -> LEQ/2(srcA)
  Variable Read3/1(VarOut) -> LEQ/3(srcB)
  LEQ/1(RungOut) -> OTU2/1(RungIn)
  OTU2/1(RungOut) -> Rung Terminal3/1(RungIn)
  XIO1/1(RungOut) -> MOV/1(RungIn)
  MOV/1(RungOut) -> TON/1(RungIn)
  Variable Read4/1(VarOut) -> MOV/2(src)
  MOV/2(dest) -> Variable Write/1(VarIn)
  MOV1/2(dest) -> Variable Write1/1(VarIn)
  Variable Read5/1(VarOut) -> MOV1/2(src)
  Variable Read6/1(VarOut) -> MOV2/2(src)
  MOV2/2(dest) -> Variable Write2/1(VarIn)
  Variable Read7/1(VarOut) -> DIV/2(srcA)
  Variable Read8/1(VarOut) -> DIV/3(srcB)
  DIV/2(dest) -> Variable Write3/1(VarIn)
  OTL1/1(RungOut) -> OTU1/1(RungIn)
  DIV/1(RungOut) -> Rung Terminal5/1(RungIn)
  TON/1(EN) -> Rung Terminal2/1(RungIn)
  Power Rail Start/1(PowerOut) -> Power Rail Terminal/1(PowerIn), XIO/1(RungIn), XIC3/1(RungIn), XIC/1(RungIn), XIC4/1(RungIn), XIC1/1(RungIn), DIV/1(RungIn)

== system Lab2_simulink_plc/PLC Controller Suite/__Logic/Temp sampling/Temperature sampling/__Logic ==
  Add Rungs : Subsystem  DisableCoverage=on  TreatAsAtomicUnit=on  mask{PLCRungNumberToAdd=2, PLCBlockType=AddRungs}
      mask prompts: PLCRungNumberToAdd "Rung Number:"
      mask Help: web([docroot '/plccoder/ref/addrungs' '.html']);
      mask DialogControl PLCAddRungs Prompt: Add Rungs
      mask DialogControl PLCRungAddButton Prompt: Add
      mask DialogControl PLCRungAddButton Callback: slplc.callbacks.addLadderRungs(gcb);
      mask Display: disp(sprintf(['Add' newline 'Rungs']));
  Add Single Rung : Subsystem  DisableCoverage=on  TreatAsAtomicUnit=on  mask{PLCRungNumberToAdd=1, PLCBlockType=AddSingleRung}
      settings: OpenFcn=slplc.callbacks.addLadderRungs(gcb);
      mask Help: web([docroot '/plccoder/ref/addsinglerung' '.html']);
      mask DialogControl PLCAddRung Prompt: Add Single Rung
      mask Display: disp(sprintf(['Add' newline '1 Rung']));
  MOV : Subsystem  Priority=6  TreatAsAtomicUnit=on  mask{PLCBlockType=MOV, PLCPOUType=stdFC}
      mask Help: web([docroot '/plccoder/ref/mov' '.html']);
      mask DialogControl PLCBlockTitle Prompt: Move (MOV)
      mask DialogControl PLCBlockDesc Prompt: Move a copy of the Source to the Destination. The Source remains unchanged.
      mask Display: disp('MOV');
  OTL : Subsystem  Priority=8  TreatAsAtomicUnit=on  mask{PLCOperandTag=TempPolling, PLCBlockType=OTL, PLCPOUType=Coil, PLCOperandExpression=xxx_PLC_VAR_TempPolling}
      mask prompts: PLCOperandTag "Operand Tag:"
      mask init:
      | slplc.callbacks.initInstruction(gcb);
      settings: AttributesFormatString=%<PLCOperandTag>
      mask Help: web([docroot '/plccoder/ref/otl' '.html']);
      mask DialogControl PLCBlockTitle Prompt: Output Latch (OTL)
      mask Display: disp('L');
  Power Rail Start : Subsystem  Priority=1  DisableCoverage=on  TreatAsAtomicUnit=on  mask{PLCBlockType=PowerRailStart}
      mask Help: web([docroot '/plccoder/ref/powerrailstart' '.html']);
      mask DialogControl PLCBlockTitle Prompt: Power Rail Start
      mask DialogControl PLCBlockDesc Prompt: This is the ladder diagram left power rail start block.
  Power Rail Terminal : Subsystem  DisableCoverage=on  TreatAsAtomicUnit=on  mask{PLCBlockType=PowerRailTerminal}
      mask Help: web([docroot '/plccoder/ref/powerrailterminal' '.html']);
      mask DialogControl PLCBlockTitle Prompt: Power Rail Terminal
      mask DialogControl PLCBlockDesc Prompt: This is the ladder diagram left power rail terminal block.
  Program Variables : Subsystem  Tag=ProgramVariableSS  DisableCoverage=on  TreatAsAtomicUnit=on  mask{PLCVartemp_periodicityName=temp_periodicity, PLCVartemp_periodicityScope=Local, PLCVartemp_periodicityPortType=Hidden, PLCVartemp_periodicityPortIndex=4, PLCVartemp_periodicityDataType=Bus: TIMER, PLCVartemp_periodicitySize=1, PLCVartemp_periodicityInitialValue=temp_period, PLCVartemp_periodicityToDelete=off, PLCVarTempPollingName=TempPolling, PLCVarTempPollingScope=External, PLCVarTempPollingPortType=Hidden, PLCVarTempPollingPortIndex=4, PLCVarTempPollingDataType=DINT, PLCVarTempPollingSize=-1, PLCVarTempPollingInitialValue=0, PLCVarTempPollingToDelete=off, PLCVarTemp_sensorName=Temp_sensor, PLCVarTemp_sensorScope=External, PLCVarTemp_sensorPortType=Hidden, PLCVarTemp_sensorPortIndex=4, PLCVarTemp_sensorDataType=DINT, PLCVarTemp_sensorSize=-1, PLCVarTemp_sensorInitialValue=0, PLCVarTemp_sensorToDelete=off, PLCVartemp_sensor_copyName=temp_sensor_copy, PLCVartemp_sensor_copyScope=External, PLCVartemp_sensor_copyPortType=Hidden, PLCVartemp_sensor_copyPortIndex=4, PLCVartemp_sensor_copyDataType=DINT, PLCVartemp_sensor_copySize=-1, PLCVartemp_sensor_copyInitialValue=0, PLCVartemp_sensor_copyToDelete=off}
      mask init:
      | slplc.callbacks.initPOUVarSS(gcb);
      settings: OpenFcn=slplc.callbacks.openProgramVariableSS(gcb);
      mask Help: web([docroot '/plccoder/ref/programvariables' '.html']);
      mask TypeOptions PLCVartemp_periodicityScope: Local | External
      mask Callback PLCVartemp_periodicityScope: slplc.callbacks.updatePOUVarSS(gcb);
      mask TypeOptions PLCVartemp_periodicityPortType: Hidden | Inport | Outport
      mask Callback PLCVartemp_periodicityPortType: slplc.callbacks.updatePOUVarSS(gcb);
      mask TypeOptions PLCVartemp_periodicityPortIndex: 1 | 2 | 3 | 4
      mask TypeOptions PLCVarTempPollingScope: Local | External
      mask Callback PLCVarTempPollingScope: slplc.callbacks.updatePOUVarSS(gcb);
      mask TypeOptions PLCVarTempPollingPortType: Hidden | Inport | Outport
      mask Callback PLCVarTempPollingPortType: slplc.callbacks.updatePOUVarSS(gcb);
      mask TypeOptions PLCVarTempPollingPortIndex: 1 | 2 | 3 | 4
      mask TypeOptions PLCVarTemp_sensorScope: Local | External
      mask Callback PLCVarTemp_sensorScope: slplc.callbacks.updatePOUVarSS(gcb);
      mask TypeOptions PLCVarTemp_sensorPortType: Hidden | Inport | Outport
      mask Callback PLCVarTemp_sensorPortType: slplc.callbacks.updatePOUVarSS(gcb);
      mask TypeOptions PLCVarTemp_sensorPortIndex: 1 | 2 | 3 | 4
      mask TypeOptions PLCVartemp_sensor_copyScope: Local | External
      mask Callback PLCVartemp_sensor_copyScope: slplc.callbacks.updatePOUVarSS(gcb);
      mask TypeOptions PLCVartemp_sensor_copyPortType: Hidden | Inport | Outport
      mask Callback PLCVartemp_sensor_copyPortType: slplc.callbacks.updatePOUVarSS(gcb);
      mask TypeOptions PLCVartemp_sensor_copyPortIndex: 1 | 2 | 3 | 4
      mask DialogControl PLCBlockTitle Prompt: Program Variable Spreadsheet
      mask DialogControl PLCVarListHeaderName Prompt: Name
      mask DialogControl PLCVarListHeaderScope Prompt: Scope
      mask DialogControl PLCVarListHeaderDataType Prompt: Data Type
      mask DialogControl PLCVarListHeaderSize Prompt: Size
      mask DialogControl PLCVarListHeaderInitialValue Prompt: Initial Value
      mask DialogControl PLCVarListHeaderToDelete Prompt: Delete
  RES : Subsystem  Tag=B  Priority=9  TreatAsAtomicUnit=on  mask{PLCOperandTag=temp_periodicity, PLCTagDataType=TIMER, PLCBlockType=RES, PLCPOUType=stdFC, PLCOperandExpression=xxx_PLC_VAR_temp_periodicity}
      mask prompts: PLCOperandTag "Operand Tag To Reset:"; PLCTagDataType "Tag Structure:"
      mask init: same as Lab2_simulink_plc/PLC Controller Suite/__Logic/Fukt sampling/Fuktmätare sampling/__Logic/RES
      settings: AttributesFormatString=%<PLCBlockType>: %<PLCOperandTag>
      mask Help: web([docroot '/plccoder/ref/res' '.html']);
      mask TypeOptions PLCTagDataType: TIMER | COUNTER | CONTROL
      mask DialogControl PLCBlockTitle Prompt: Reset TIMER, COUNTER, or CONTROL structure (RES)
      mask Display: disp(['RES'  newline newline 'temp_periodicity']);
  Rung Terminal1 : Subsystem  Priority=10  DisableCoverage=on  TreatAsAtomicUnit=on  mask{PLCRungTerminalIndex=2, PLCBlockType=RungTerminal}
      settings: AttributesFormatString=Rung %<PLCRungTerminalIndex>
      mask Help: web([docroot '/plccoder/ref/rungterminal' '.html']);
      mask DialogControl PLCBlockTitle Prompt: Rung Terminal
      mask DialogControl PLCBlockDescText Prompt: This is the ladder diagram rung terminal.
  Rung Terminal2 : Subsystem  Priority=3  DisableCoverage=on  TreatAsAtomicUnit=on  mask{PLCRungTerminalIndex=1, PLCBlockType=RungTerminal}
      settings: AttributesFormatString=Rung %<PLCRungTerminalIndex>
      mask Help: web([docroot '/plccoder/ref/rungterminal' '.html']);
      mask DialogControl PLCBlockTitle Prompt: Rung Terminal
      mask DialogControl PLCBlockDescText Prompt: This is the ladder diagram rung terminal.
  TON : Subsystem  Priority=2  TreatAsAtomicUnit=on  mask{PLCOperandTag=temp_periodicity, PLCTimerSampleTime=0.2, PLCBlockType=TON, PLCPOUType=stdFB, PLCOperandAccess=ReadWrite, PLCOperandExpression=xxx_PLC_VAR_temp_periodicity}
      mask prompts: PLCOperandTag "Timer Tag:"; PLCTimerSampleTime "Timer Sample Time:"
      mask init:
      | block = gcb;
      | maskObj = Simulink.Mask.get(block);
      | maskObj.Display = sprintf('disp([''%s'' newline newline ''%s'']);', PLCBlockType, PLCOperandTag);
      | slplc.callbacks.initInstruction(block);
      settings: AttributesFormatString=%<PLCBlockType>: %<PLCOperandTag>
      mask Help: web([docroot '/plccoder/ref/ton' '.html']);
      mask DialogControl PLCBlockTitle Prompt: Timer On Delay (TON)
      mask Display: disp(['TON' newline newline 'temp_periodicity']);
  Up to Parent POU : Subsystem  DisableCoverage=on  TreatAsAtomicUnit=on  mask{PLCBlockType=OpenParentPOU}
      settings: OpenFcn=slplc.callbacks.openParentPOUBlock(gcb);
      mask Help: web([docroot '/plccoder/ref/uptoparentpou' '.html']);
      mask DialogControl PLCBlockTitle Prompt: Add Single Rung
  Variable Read : Subsystem  Priority=5  TreatAsAtomicUnit=on  mask{PLCOperandTag=Temp_sensor, PLCBlockType=VariableRead, PLCOperandAccess=Read, PLCPOUType=VariableRead, PLCOperandExpression=xxx_PLC_VAR_Temp_sensor}
      mask prompts: PLCOperandTag "Data Tag:"
      mask init:
      | block = gcb;
      | maskObj = Simulink.Mask.get(block);
      | maskObj.Display = sprintf('disp(''%s'');', PLCOperandTag);
      | slplc.callbacks.initInstruction(block);
      mask Help: web([docroot '/plccoder/ref/variableread' '.html']);
      mask DialogControl PLCBlockTitle Prompt: Data Tag Read
      mask Display: disp('Temp_sensor');
  Variable Write : Subsystem  Priority=7  TreatAsAtomicUnit=on  mask{PLCOperandTag=temp_sensor_copy, PLCBlockType=VariableWrite, PLCOperandAccess=Write, PLCPOUType=VariableWrite, PLCOperandExpression=xxx_PLC_VAR_temp_sensor_copy}
      mask prompts: PLCOperandTag "Data Tag:"
      mask init: same as Lab2_simulink_plc/PLC Controller Suite/__Logic/Fukt logic/Program/__Logic/Variable Write
      mask Help: web([docroot '/plccoder/ref/variablewrite' '.html']);
      mask DialogControl PLCBlockTitle Prompt: Data Tag Write
      mask Display: disp('temp_sensor_copy');
  XIC : Subsystem  Priority=4  TreatAsAtomicUnit=on  mask{PLCOperandTag=temp_periodicity.DN, PLCBlockType=XIC, PLCPOUType=Contact, PLCOperandExpression=xxx_PLC_VAR_temp_periodicity.DN}
      mask prompts: PLCOperandTag "Operand Tag:"
      mask init:
      | slplc.callbacks.initInstruction(gcb);
      settings: AttributesFormatString=%<PLCOperandTag>
      mask Help: web([docroot '/plccoder/ref/xic' '.html']);
      mask DialogControl PLCBlockTitle Prompt: Examine If Closed (XIC)
  wires:
  Power Rail Start/1(PowerOut) -> XIC/1(RungIn), Power Rail Terminal/1(PowerIn), TON/1(RungIn)
  Variable Read/1(VarOut) -> MOV/2(src)
  MOV/2(dest) -> Variable Write/1(VarIn)
  MOV/1(RungOut) -> OTL/1(RungIn)
  OTL/1(RungOut) -> RES/1(RungIn)
  TON/1(EN) -> Rung Terminal2/1(RungIn)
  XIC/1(RungOut) -> MOV/1(RungIn)
  RES/1(RungOut) -> Rung Terminal1/1(RungIn)
